simulink library "Renault_Library"  (Simulink 8.2)
settings: Solver=ode3  StartTime=0.0  StopTime=10.0  FixedStep=0.020  RelTol=1e-3
undefined symbols (not defined in the file or in its folder): ABC, AssystemProposal, b, chkval, CoeffA_C, CoeffB_C, CoeffMean_C, ConfTime_C, DiagDelay_C, DividePercent, FailureConfActive_C, FallingEdgeDuration_C, HighLevelThresholdVoltage_C, HighLevelVoltage_C, HighLevelVoltageTunable_C, InitValue_C, InputVoltRangeMax_C, InputVoltRangeMin_C, LowActiveContact_C, LowLevelThresholdVoltage_C, LowLevelVoltage_C, MaxOperatingVoltage_C, MinOperatingVoltage_C, mV2V_conversion, OUT1, OutOfRangeHigh_C, OutOfRangeLow_C, PinSupplySaturationMax, PinSupplySaturationMin, Res1Value_C, Res2Value_C, Res3Value_C, RisingEdgeDuration_C, RSA_CANSignalFailMonitoring, SampleTime_C, service14Table, service19Table, service22Table, service24Table, service28Table, service2ETable, service2FTable, service31Table, service87Table, SizeXStateDID2FTable, SizeXTable14, SizeXTable19, SizeXTable22, SizeXTable24, SizeXTable28, SizeXTable2E, SizeXTable2F, SizeXTable31, SizeXTable87, SizeYStateDID2FTable, SizeYTable14, SizeYTable19, SizeYTable22, SizeYTable24, SizeYTable28 ... (+22)
undefined symbols (not defined in the file): BatteryVoltage_ActivationThreshold_C, FrameStatus_AbsentValue_C, FrameStatus_ConfAbsentValue_C, MemThreshold_C, NAMEOFTHEMODEL_SAMPLE_TIME_CST, NbAbs1_C, NbConfAbs1_C, NbConfPres1_C, NbPres1_C, NOMDUSIGNAL_FaultConfTime_C, NOMDUSIGNAL_FaultRehabTime_C, NOMDUSIGNAL_switchvalue_C, NOMDUSIGNAL_unavailablevalue_C, PeriodFrame1_C, ResetTime_C, WakeUpTime_C
libraries: Renault_Library x112, simulink x75
(part 13 of 32)

== system Renault_Library/Services ECU/Diag/RSA_DiagnosticManagement_v02.00 ==
  DTOOL_TO_ECU_RECEIVED : Inport  OutDataTypeStr=uint8
  PART1_DTOOL_TO_ECU : Inport  Port=2  OutDataTypeStr=uint32
  PART2_DTOOL_TO_ECU : Inport  Port=3  OutDataTypeStr=uint32
  DIAG_INPUT : Inport  Port=4  OutDataTypeStr=Bus: busInput
  DIAGNOSTIC_ACTION_TOP : Subsystem
  DIAGNOSTIC_SANCTION_TOP : Subsystem
  DIAGNOSTIC_SEGMENTATION_TOP : Subsystem
  ECU_TO_DTOOL_RECEIVED : Outport  OutDataTypeStr=uint8
  PART1_ECU_TO_DTOOL : Outport  Port=2  OutDataTypeStr=uint32
  PART2_ECU_TO_DTOOL : Outport  Port=3  OutDataTypeStr=uint32
  DIAG_OUTPUT : Outport  Port=4  OutDataTypeStr=Bus: busOutput
  wires:
  DIAGNOSTIC_SEGMENTATION_TOP/2(PART1_ECU_TO_DTOOL) -> PART1_ECU_TO_DTOOL/1
  DIAGNOSTIC_SEGMENTATION_TOP/3(PART2_ECU_TO_DTOOL) -> PART2_ECU_TO_DTOOL/1
  DIAGNOSTIC_SEGMENTATION_TOP/1(ECU_TO_DTOOL_SEND) -> ECU_TO_DTOOL_RECEIVED/1
  [DIAG_BUFFER_INPUT] -> DIAGNOSTIC_SEGMENTATION_TOP/4(DIAG_BUFFER_RESPONSE)
  DIAGNOSTIC_ACTION_TOP/2(DIAG_BUFFER_RESPONSE) -> [DIAG_BUFFER_INPUT]
  DTOOL_TO_ECU_RECEIVED/1 -> DIAGNOSTIC_SEGMENTATION_TOP/1(DTOOL_TO_ECU_FRAME_RECEIVED)
  PART1_DTOOL_TO_ECU/1 -> DIAGNOSTIC_SEGMENTATION_TOP/2(PART1_DTOOL_TO_ECU)
  PART2_DTOOL_TO_ECU/1 -> DIAGNOSTIC_SEGMENTATION_TOP/3(PART2_DTOOL_TO_ECU)
  DIAG_INPUT/1 -> DIAGNOSTIC_ACTION_TOP/6(DIAG_INPUT)
  DIAGNOSTIC_ACTION_TOP/3(RES_DATA_NUMBER) -> [RES_DATA_NUMBER]
  [RES_DATA_NUMBER] -> DIAGNOSTIC_SEGMENTATION_TOP/5(RES_DATA_NUMBER)
  DIAGNOSTIC_SEGMENTATION_TOP/5(REQ_DATA_NUMBER) -> DIAGNOSTIC_SANCTION_TOP/2(REQ_DATA_NUMBER)
  DIAGNOSTIC_SEGMENTATION_TOP/6(SEG_STATE) -> DIAGNOSTIC_SANCTION_TOP/3(SEG_STATE)
  DIAGNOSTIC_SANCTION_TOP/1(NEGATIVE_RES_CODE) -> DIAGNOSTIC_ACTION_TOP/2(NEGATIVE_RES_CODE)
  DIAGNOSTIC_SANCTION_TOP/2(SUPPRESS_POS_RES) -> DIAGNOSTIC_ACTION_TOP/3(SUPPRESS_POS_RES)
  DIAGNOSTIC_SANCTION_TOP/3(SEND_RES_MESSAGE) -> DIAGNOSTIC_ACTION_TOP/4(SEND_RES_MESSAGE)
  DIAGNOSTIC_SANCTION_TOP/4(LIST_DID_SERVICE_22) -> DIAGNOSTIC_ACTION_TOP/5(LIST_DID_SERVICE_22)
  DIAGNOSTIC_SEGMENTATION_TOP/4(DIAG_BUFFER_REQUEST) -> DIAGNOSTIC_ACTION_TOP/1(DIAG_BUFFER_REQUEST), DIAGNOSTIC_SANCTION_TOP/1(DIAG_BUFFER_REQUEST)
  DIAGNOSTIC_ACTION_TOP/1(DIAG_OUTPUT) -> DIAG_OUTPUT/1

== system Renault_Library/Services ECU/Diag/RSA_FailureManagementSwitch ==
  FunctionalSignal : Inport
  Vbat : Inport  Port=2
  SignalFailure : Inport  Port=3
  SignalValue : Inport  Port=4
  SignalApplyValue : Inport  Port=5
  SignalFailureType : Inport  Port=6
  GroundValue : Constant  Value=GroundValue
  MultiportSwitch : MultiPortSwitch  Inputs=6  RndMeth=Zero  InputSameDT=on
  OpenCircuitValue : Constant  Value=OpenCircuitValue
  Switch : Switch  Criteria=u2 ~= 0  Threshold=0.5  RndMeth=Zero  InputSameDT=on
  Switch1 : Switch  Criteria=u2 ~= 0  Threshold=0.5  RndMeth=Zero  InputSameDT=on
  UnitDelay : UnitDelay  SampleTime=-1
  UnitDelay1 : UnitDelay  SampleTime=-1
  SignalOut : Outport
  wires:
  MultiportSwitch/1 -> Switch1/1
  SignalFailureType/1 -> MultiportSwitch/1
  Switch/1 -> UnitDelay/1, MultiportSwitch/4
  UnitDelay/1 -> Switch/3
  SignalFailure/1 -> Switch1/2
  FunctionalSignal/1 -> [FunctionalSignal]
  [FunctionalSignal] -> Switch1/3
  Switch1/1 -> SignalOut/1, [SignalOut]
  [FunctionalSignal] -> MultiportSwitch/2
  SignalValue/1 -> Switch/1
  [SignalOut] -> UnitDelay1/1
  UnitDelay1/1 -> MultiportSwitch/3
  GroundValue/1 -> MultiportSwitch/5
  OpenCircuitValue/1 -> MultiportSwitch/7
  Vbat/1 -> MultiportSwitch/6
  SignalApplyValue/1 -> Switch/2

== system Renault_Library/Services ECU/EEPROM/RSA_UpdateEEPROMData ==
  DATA_MODEL_EEPROM : Inport
      settings: AttributesFormatString=Type : %<OutDataTypeStr>
  DATA_STORE_READ : Inport  Port=2
      settings: AttributesFormatString=Type : %<OutDataTypeStr>
  RelationalOperator : RelationalOperator  Operator=~=  OutDataTypeStr=boolean  InputSameDT=on
  Switch : Switch  Criteria=u2 ~= 0  RndMeth=Zero  InputSameDT=on
      settings: AttributesFormatString=Threshold : %<Threshold>\nSaturation:%<SaturateOnIntegerOverflow>\nCriteria:%<Criteria>
  UnitDelay : UnitDelay  SampleTime=-1
      settings: AttributesFormatString=Initial value : %<X0>
  DATA_STORE_WRITE : Outport  OutputWhenDisabled=reset  InitialOutput=0
      settings: AttributesFormatString=Type : %<OutDataTypeStr>\nInit :%<InitialOutput>
  wires:
  DATA_MODEL_EEPROM/1 -> RelationalOperator/2, Switch/1, UnitDelay/1
  UnitDelay/1 -> RelationalOperator/1
  RelationalOperator/1 -> Switch/2
  DATA_STORE_READ/1 -> Switch/3
  Switch/1 -> DATA_STORE_WRITE/1

== system Renault_Library/Services ECU/HW Inputs/RSA_CHA8PRO ==
  note: cf. Modeling_Requirements_36-00-002_v1.2.doc
      | Requirements linked to this component:
      | RQT_LOGIN_GEN001_CHA8PRO
      | RQT_LOGIN_GEN002_CHA8PRO
      | RQT_LOGIN_GEN003_CHA8PRO
      | RQT_LOGIN_GEN004_CHA8PRO
      | RQT_LOGIN_GEN005_CHA8PRO
      | RQT_LOGIN_GEN006_CHA8PRO
      | RQT_LOGIN_GEN007_CHA8PRO
      | RQT_LOGIN_GEN008_CHA8PRO
  SwitchVoltage : Inport
  SupplyVoltage : Inport  Port=2
  RSA_ServECU_Activation : Renault_Library/Services ECU/Commonly_Used_Blocks/RSA_ServECU_Activation
  RSA_ServECU_EnabledSwitch : Renault_Library/Services ECU/Commonly_Used_Blocks/RSA_ServECU_EnabledSwitch
  Terminator : Terminator
  LogicalState_F : Outport  OutputWhenDisabled=reset  InitialOutput=0
  wires:
  SwitchVoltage/1 -> RSA_ServECU_EnabledSwitch/1
  RSA_ServECU_EnabledSwitch/1 -> Terminator/1
  RSA_ServECU_EnabledSwitch/2 -> LogicalState_F/1
  SupplyVoltage/1 -> RSA_ServECU_Activation/1
  RSA_ServECU_Activation/1 -> RSA_ServECU_EnabledSwitch/Enable

== system Renault_Library/Services ECU/HW Inputs/RSA_CHB6 ==
  note: cf. Modeling_Requirements_36-00-002_v1.2.doc
      | Requirements linked to this component:
      | RQT_LOGIN_GEN001_CHB6
      | RQT_LOGIN_GEN002_CHB6
      | RQT_LOGIN_GEN003_CHB6
      | RQT_LOGIN_GEN004_CHB6
      | RQT_LOGIN_GEN005_CHB6
      | RQT_LOGIN_GEN006_CHB6
      | RQT_LOGIN_GEN007_CHB6
      | RQT_LOGIN_GEN008_CHB6
  SwitchVoltage : Inport
  SupplyVoltage : Inport  Port=2
  RSA_ServECU_Activation : Renault_Library/Services ECU/Commonly_Used_Blocks/RSA_ServECU_Activation
  RSA_ServECU_EnabledSwitch : Renault_Library/Services ECU/Commonly_Used_Blocks/RSA_ServECU_EnabledSwitch
  Terminator : Terminator
  LogicalState_F : Outport  OutputWhenDisabled=reset  InitialOutput=0
  wires:
  RSA_ServECU_Activation/1 -> RSA_ServECU_EnabledSwitch/Enable
  SupplyVoltage/1 -> RSA_ServECU_Activation/1
  SwitchVoltage/1 -> RSA_ServECU_EnabledSwitch/1
  RSA_ServECU_EnabledSwitch/1 -> Terminator/1
  RSA_ServECU_EnabledSwitch/2 -> LogicalState_F/1

== system Renault_Library/Services ECU/HW Inputs/RSA_CHB6DAS ==
  note: cf. Modeling_Requirements_36-00-002_v1.2.doc
      | Requirements linked to this component:
      | RQT_LOGIN_GEN001_CHB6DAS
      | RQT_LOGIN_GEN002_CHB6DAS
      | RQT_LOGIN_GEN003_CHB6DAS
      | RQT_LOGIN_GEN004_CHB6DAS
      | RQT_LOGIN_GEN005_CHB6DAS
      | RQT_LOGIN_GEN006_CHB6DAS
      | RQT_LOGIN_GEN007_CHB6DAS
      | RQT_LOGIN_GEN008_CHB6DAS
  SwitchVoltage : Inport
  SupplyVoltage : Inport  Port=2
  RSA_ServECU_Activation : Renault_Library/Services ECU/Commonly_Used_Blocks/RSA_ServECU_Activation
  RSA_ServECU_EnabledSwitch : Renault_Library/Services ECU/Commonly_Used_Blocks/RSA_ServECU_EnabledSwitch
  Terminator : Terminator
  LogicalState_F : Outport  OutputWhenDisabled=reset  InitialOutput=0
  wires:
  SwitchVoltage/1 -> RSA_ServECU_EnabledSwitch/1
  RSA_ServECU_EnabledSwitch/1 -> Terminator/1
  RSA_ServECU_EnabledSwitch/2 -> LogicalState_F/1
  RSA_ServECU_Activation/1 -> RSA_ServECU_EnabledSwitch/Enable
  SupplyVoltage/1 -> RSA_ServECU_Activation/1

== system Renault_Library/Services ECU/HW Inputs/RSA_CHXX ==
  note: cf. Modeling_Requirements_36-00-002_v1.2.doc
      | Requirements linked to this component:
      | RQT_LOGIN_GEN001_CHXX
      | RQT_LOGIN_GEN002_CHXX
      | RQT_LOGIN_GEN003_CHXX
      | RQT_LOGIN_GEN004_CHXX
      | RQT_LOGIN_GEN005_CHXX
      | RQT_LOGIN_GEN006_CHXX
      | RQT_LOGIN_GEN007_CHXX
      | RQT_LOGIN_GEN008_CHXX
  SwitchVoltage : Inport
  SupplyVoltage : Inport  Port=2
  RSA_ServECU_Activation : Renault_Library/Services ECU/Commonly_Used_Blocks/RSA_ServECU_Activation
  RSA_ServECU_EnabledSwitch : Renault_Library/Services ECU/Commonly_Used_Blocks/RSA_ServECU_EnabledSwitch
  Terminator : Terminator
  LogicalState_F : Outport  OutputWhenDisabled=reset  InitialOutput=0
  wires:
  RSA_ServECU_EnabledSwitch/2 -> LogicalState_F/1
  RSA_ServECU_EnabledSwitch/1 -> Terminator/1
  SwitchVoltage/1 -> RSA_ServECU_EnabledSwitch/1
  SupplyVoltage/1 -> RSA_ServECU_Activation/1
  RSA_ServECU_Activation/1 -> RSA_ServECU_EnabledSwitch/Enable

== system Renault_Library/Services ECU/HW Inputs/RSA_CLA8LOCK ==
  note: cf. Modeling_Requirements_36-00-002_v1.2.doc
      | Requirements linked to this component:
      | RQT_ALOGIN_GEN001_CLA8LOCK
      | RQT_ALOGIN_GEN002_CLA8LOCK
      | RQT_ALOGIN_GEN003_CLA8LOCK
      | RQT_ALOGIN_GEN004_CLA8LOCK
      | RQT_ALOGIN_GEN005_CLA8LOCK
      | RQT_ALOGIN_GEN006_CLA8LOCK
      | RQT_ALOGIN_GEN007_CLA8LOCK
      | RQT_ALOGIN_GEN008_CLA8LOCK
      | RQT_ALOGIN_GEN009_CLA8LOCK
  SwitchVoltage : Inport
  SupplyVoltage : Inport  Port=2
  RSA_ServECU_Activation : Renault_Library/Services ECU/Commonly_Used_Blocks/RSA_ServECU_Activation
  RSA_ServECU_EnabledInputContact : Renault_Library/Services ECU/Commonly_Used_Blocks/RSA_ServECU_EnabledInputContact
  Terminator : Terminator
  SwitchState_F : Outport
  wires:
  SwitchVoltage/1 -> RSA_ServECU_EnabledInputContact/1
  SupplyVoltage/1 -> RSA_ServECU_Activation/1
  RSA_ServECU_EnabledInputContact/1 -> Terminator/1
  RSA_ServECU_EnabledInputContact/2 -> SwitchState_F/1
  RSA_ServECU_Activation/1 -> RSA_ServECU_EnabledInputContact/Enable

== system Renault_Library/Services ECU/HW Inputs/RSA_CLA8MVAC ==
  note: cf. Modeling_Requirements_36-00-002_v1.2.doc
      | Requirements linked to this component:
      | RQT_ALOGIN_GEN001_CLA8MVAC
      | RQT_ALOGIN_GEN002_CLA8MVAC
      | RQT_ALOGIN_GEN003_CLA8MVAC
      | RQT_ALOGIN_GEN004_CLA8MVAC
      | RQT_ALOGIN_GEN005_CLA8MVAC
      | RQT_ALOGIN_GEN006_CLA8MVAC
      | RQT_ALOGIN_GEN007_CLA8MVAC
      | RQT_ALOGIN_GEN008_CLA8MVAC
      | RQT_ALOGIN_GEN009_CLA8MVAC
  SwitchVoltage : Inport
  SupplyVoltage : Inport  Port=2
  RSA_ServECU_Activation : Renault_Library/Services ECU/Commonly_Used_Blocks/RSA_ServECU_Activation
  RSA_ServECU_EnabledInputContact : Renault_Library/Services ECU/Commonly_Used_Blocks/RSA_ServECU_EnabledInputContact
  Terminator : Terminator
  SwitchState_F : Outport
  wires:
  RSA_ServECU_Activation/1 -> RSA_ServECU_EnabledInputContact/Enable
  RSA_ServECU_EnabledInputContact/2 -> SwitchState_F/1
  RSA_ServECU_EnabledInputContact/1 -> Terminator/1
  SupplyVoltage/1 -> RSA_ServECU_Activation/1
  SwitchVoltage/1 -> RSA_ServECU_EnabledInputContact/1

== system Renault_Library/Services ECU/HW Inputs/RSA_CLA8PRO ==
  note: cf. Modeling_Requirements_36-00-002_v1.2.doc
      | Requirements linked to this component:
      | RQT_LOGIN_GEN001_CLA8PRO
      | RQT_LOGIN_GEN002_CLA8PRO
      | RQT_LOGIN_GEN003_CLA8PRO
      | RQT_LOGIN_GEN004_CLA8PRO
      | RQT_LOGIN_GEN005_CLA8PRO
      | RQT_LOGIN_GEN006_CLA8PRO
      | RQT_LOGIN_GEN007_CLA8PRO
      | RQT_LOGIN_GEN008_CLA8PRO
  SwitchVoltage : Inport
  SupplyVoltage : Inport  Port=2
  RSA_ServECU_Activation : Renault_Library/Services ECU/Commonly_Used_Blocks/RSA_ServECU_Activation
  RSA_ServECU_EnabledSwitch : Renault_Library/Services ECU/Commonly_Used_Blocks/RSA_ServECU_EnabledSwitch
  Terminator : Terminator
  LogicalState_F : Outport  OutputWhenDisabled=reset  InitialOutput=0
  wires:
  SupplyVoltage/1 -> RSA_ServECU_Activation/1
  SwitchVoltage/1 -> RSA_ServECU_EnabledSwitch/1
  RSA_ServECU_Activation/1 -> RSA_ServECU_EnabledSwitch/Enable
  RSA_ServECU_EnabledSwitch/1 -> Terminator/1
  RSA_ServECU_EnabledSwitch/2 -> LogicalState_F/1

== system Renault_Library/Services ECU/HW Inputs/RSA_CLA8SEAT ==
  note: cf. Modeling_Requirements_36-00-002_v1.2.doc
      | Requirements linked to this component:
      | RQT_ALOGIN_GEN001_CLA8SEAT
      | RQT_ALOGIN_GEN002_CLA8SEAT
      | RQT_ALOGIN_GEN003_CLA8SEAT
      | RQT_ALOGIN_GEN004_CLA8SEAT
      | RQT_ALOGIN_GEN005_CLA8SEAT
      | RQT_ALOGIN_GEN006_CLA8SEAT
      | RQT_ALOGIN_GEN007_CLA8SEAT
      | RQT_ALOGIN_GEN008_CLA8SEAT
      | RQT_ALOGIN_GEN009_CLA8SEAT
  SwitchVoltage : Inport
  SupplyVoltage : Inport  Port=2
  RSA_ServECU_Activation : Renault_Library/Services ECU/Commonly_Used_Blocks/RSA_ServECU_Activation
  RSA_ServECU_EnabledInputContact : Renault_Library/Services ECU/Commonly_Used_Blocks/RSA_ServECU_EnabledInputContact
  Terminator : Terminator
  SwitchState_F : Outport
  wires:
  RSA_ServECU_Activation/1 -> RSA_ServECU_EnabledInputContact/Enable
  RSA_ServECU_EnabledInputContact/2 -> SwitchState_F/1
  RSA_ServECU_EnabledInputContact/1 -> Terminator/1
  SupplyVoltage/1 -> RSA_ServECU_Activation/1
  SwitchVoltage/1 -> RSA_ServECU_EnabledInputContact/1

== system Renault_Library/Services ECU/HW Inputs/RSA_CLA8WAS ==
  note: cf. Modeling_Requirements_36-00-002_v1.2.doc
      | Requirements linked to this component:
      | RQT_LOGIN_GEN001_CLA8WAS
      | RQT_LOGIN_GEN002_CLA8WAS
      | RQT_LOGIN_GEN003_CLA8WAS
      | RQT_LOGIN_GEN004_CLA8WAS
      | RQT_LOGIN_GEN005_CLA8WAS
      | RQT_LOGIN_GEN006_CLA8WAS
      | RQT_LOGIN_GEN007_CLA8WAS
      | RQT_LOGIN_GEN008_CLA8WAS
  SwitchVoltage : Inport
  SupplyVoltage : Inport  Port=2
  RSA_ServECU_Activation : Renault_Library/Services ECU/Commonly_Used_Blocks/RSA_ServECU_Activation
  RSA_ServECU_EnabledSwitch : Renault_Library/Services ECU/Commonly_Used_Blocks/RSA_ServECU_EnabledSwitch
  Terminator : Terminator
  LogicalState_F : Outport  OutputWhenDisabled=reset  InitialOutput=0
  wires:
  RSA_ServECU_EnabledSwitch/2 -> LogicalState_F/1
  RSA_ServECU_EnabledSwitch/1 -> Terminator/1
  RSA_ServECU_Activation/1 -> RSA_ServECU_EnabledSwitch/Enable
  SupplyVoltage/1 -> RSA_ServECU_Activation/1
  SwitchVoltage/1 -> RSA_ServECU_EnabledSwitch/1

== system Renault_Library/Services ECU/HW Inputs/RSA_CLB6 ==
  note: cf. Modeling_Requirements_36-00-002_v1.2.doc
      | Requirements linked to this component:
      | RQT_LOGIN_GEN001_CLB6
      | RQT_LOGIN_GEN002_CLB6
      | RQT_LOGIN_GEN003_CLB6
      | RQT_LOGIN_GEN004_CLB6
      | RQT_LOGIN_GEN005_CLB6
      | RQT_LOGIN_GEN006_CLB6
      | RQT_LOGIN_GEN007_CLB6
      | RQT_LOGIN_GEN008_CLB6
  SwitchVoltage : Inport
  SupplyVoltage : Inport  Port=2
  RSA_ServECU_Activation : Renault_Library/Services ECU/Commonly_Used_Blocks/RSA_ServECU_Activation
  RSA_ServECU_EnabledSwitch : Renault_Library/Services ECU/Commonly_Used_Blocks/RSA_ServECU_EnabledSwitch
  Terminator : Terminator
  LogicalState_F : Outport  OutputWhenDisabled=reset  InitialOutput=0
  wires:
  RSA_ServECU_EnabledSwitch/2 -> LogicalState_F/1
  RSA_ServECU_EnabledSwitch/1 -> Terminator/1
  RSA_ServECU_Activation/1 -> RSA_ServECU_EnabledSwitch/Enable
  SwitchVoltage/1 -> RSA_ServECU_EnabledSwitch/1
  SupplyVoltage/1 -> RSA_ServECU_Activation/1

== system Renault_Library/Services ECU/HW Inputs/RSA_CLB6DAS ==
  note: cf. Modeling_Requirements_36-00-002_v1.2.doc
      | Requirements linked to this component:
      | RQT_LOGIN_GEN001_CLB6DAS
      | RQT_LOGIN_GEN002_CLB6DAS
      | RQT_LOGIN_GEN003_CLB6DAS
      | RQT_LOGIN_GEN004_CLB6DAS
      | RQT_LOGIN_GEN005_CLB6DAS
      | RQT_LOGIN_GEN006_CLB6DAS
      | RQT_LOGIN_GEN007_CLB6DAS
      | RQT_LOGIN_GEN008_CLB6DAS
  SwitchVoltage : Inport
  SupplyVoltage : Inport  Port=2
  RSA_ServECU_Activation : Renault_Library/Services ECU/Commonly_Used_Blocks/RSA_ServECU_Activation
  RSA_ServECU_EnabledSwitch : Renault_Library/Services ECU/Commonly_Used_Blocks/RSA_ServECU_EnabledSwitch
  Terminator : Terminator
  LogicalState_F : Outport  OutputWhenDisabled=reset  InitialOutput=0
  wires:
  SwitchVoltage/1 -> RSA_ServECU_EnabledSwitch/1
  RSA_ServECU_EnabledSwitch/1 -> Terminator/1
  RSA_ServECU_Activation/1 -> RSA_ServECU_EnabledSwitch/Enable
  SupplyVoltage/1 -> RSA_ServECU_Activation/1
  RSA_ServECU_EnabledSwitch/2 -> LogicalState_F/1

== system Renault_Library/Services ECU/HW Inputs/RSA_CLXX ==
  note: cf. Modeling_Requirements_36-00-002_v1.2.doc
      | Requirements linked to this component:
      | RQT_LOGIN_GEN001_CLXX
      | RQT_LOGIN_GEN002_CLXX
      | RQT_LOGIN_GEN003_CLXX
      | RQT_LOGIN_GEN004_CLXX
      | RQT_LOGIN_GEN005_CLXX
      | RQT_LOGIN_GEN006_CLXX
      | RQT_LOGIN_GEN007_CLXX
      | RQT_LOGIN_GEN008_CLXX
  SwitchVoltage : Inport
  SupplyVoltage : Inport  Port=2
  RSA_ServECU_Activation : Renault_Library/Services ECU/Commonly_Used_Blocks/RSA_ServECU_Activation
  RSA_ServECU_EnabledSwitch : Renault_Library/Services ECU/Commonly_Used_Blocks/RSA_ServECU_EnabledSwitch
  Terminator : Terminator
  LogicalState_F : Outport  OutputWhenDisabled=reset  InitialOutput=0
  wires:
  SupplyVoltage/1 -> RSA_ServECU_Activation/1
  SwitchVoltage/1 -> RSA_ServECU_EnabledSwitch/1
  RSA_ServECU_Activation/1 -> RSA_ServECU_EnabledSwitch/Enable
  RSA_ServECU_EnabledSwitch/1 -> Terminator/1
  RSA_ServECU_EnabledSwitch/2 -> LogicalState_F/1

== system Renault_Library/Services ECU/HW Inputs/RSA_IHB6 ==
  note: cf. Modeling_Requirements_36-00-002_v1.2.doc
      | Requirements linked to this component:
      | RQT_LOGIN_GEN001_IHB6
      | RQT_LOGIN_GEN002_IHB6
      | RQT_LOGIN_GEN003_IHB6
      | RQT_LOGIN_GEN004_IHB6
      | RQT_LOGIN_GEN005_IHB6
      | RQT_LOGIN_GEN006_IHB6
      | RQT_LOGIN_GEN007_IHB6
      | RQT_LOGIN_GEN008_IHB6
  SwitchVoltage : Inport
  SupplyVoltage : Inport  Port=2
  RSA_ServECU_Activation : Renault_Library/Services ECU/Commonly_Used_Blocks/RSA_ServECU_Activation
  RSA_ServECU_EnabledSwitch : Renault_Library/Services ECU/Commonly_Used_Blocks/RSA_ServECU_EnabledSwitch
  Terminator : Terminator
  LogicalState_F : Outport  OutputWhenDisabled=reset  InitialOutput=0
  wires:
  SupplyVoltage/1 -> RSA_ServECU_Activation/1
  SwitchVoltage/1 -> RSA_ServECU_EnabledSwitch/1
  RSA_ServECU_Activation/1 -> RSA_ServECU_EnabledSwitch/Enable
  RSA_ServECU_EnabledSwitch/1 -> Terminator/1
  RSA_ServECU_EnabledSwitch/2 -> LogicalState_F/1

== system Renault_Library/Services ECU/HW Inputs/RSA_IHB6LED ==
  note: cf. Modeling_Requirements_36-00-002_v1.2.doc
      | Requirements linked to this component:
      | RQT_LOGIN_GEN001_IHB6LED
      | RQT_LOGIN_GEN002_IHB6LED
      | RQT_LOGIN_GEN003_IHB6LED
      | RQT_LOGIN_GEN004_IHB6LED
      | RQT_LOGIN_GEN005_IHB6LED
      | RQT_LOGIN_GEN006_IHB6LED
      | RQT_LOGIN_GEN007_IHB6LED
      | RQT_LOGIN_GEN008_IHB6LED
      | RQT_LOGIN_ADDGEN001_IHB6LED
  SwitchVoltage : Inport
  SupplyVoltage : Inport  Port=2
  RSA_ServECU_Activation : Renault_Library/Services ECU/Commonly_Used_Blocks/RSA_ServECU_Activation
  RSA_ServECU_EnabledSwitch : Renault_Library/Services ECU/Commonly_Used_Blocks/RSA_ServECU_EnabledSwitch
  LogicalState_LED : Outport  OutputWhenDisabled=reset  InitialOutput=0
  LogicalState_F : Outport  Port=2  OutputWhenDisabled=reset  InitialOutput=0
  wires:
  RSA_ServECU_EnabledSwitch/2 -> LogicalState_F/1
  RSA_ServECU_EnabledSwitch/1 -> LogicalState_LED/1
  RSA_ServECU_Activation/1 -> RSA_ServECU_EnabledSwitch/Enable
  SwitchVoltage/1 -> RSA_ServECU_EnabledSwitch/1
  SupplyVoltage/1 -> RSA_ServECU_Activation/1

== system Renault_Library/Services ECU/HW Inputs/RSA_IHXX ==
  note: cf. Modeling_Requirements_36-00-002_v1.2.doc
      | Requirements linked to this component:
      | RQT_LOGIN_GEN001_IHXX
      | RQT_LOGIN_GEN002_IHXX
      | RQT_LOGIN_GEN003_IHXX
      | RQT_LOGIN_GEN004_IHXX
      | RQT_LOGIN_GEN005_IHXX
      | RQT_LOGIN_GEN006_IHXX
      | RQT_LOGIN_GEN007_IHXX
      | RQT_LOGIN_GEN008_IHXX
  SwitchVoltage : Inport
  SupplyVoltage : Inport  Port=2
  RSA_ServECU_Activation : Renault_Library/Services ECU/Commonly_Used_Blocks/RSA_ServECU_Activation
  RSA_ServECU_EnabledSwitch : Renault_Library/Services ECU/Commonly_Used_Blocks/RSA_ServECU_EnabledSwitch
  Terminator : Terminator
  LogicalState_F : Outport  OutputWhenDisabled=reset  InitialOutput=0
  wires:
  SupplyVoltage/1 -> RSA_ServECU_Activation/1
  SwitchVoltage/1 -> RSA_ServECU_EnabledSwitch/1
  RSA_ServECU_Activation/1 -> RSA_ServECU_EnabledSwitch/Enable
  RSA_ServECU_EnabledSwitch/1 -> Terminator/1
  RSA_ServECU_EnabledSwitch/2 -> LogicalState_F/1

== system Renault_Library/Services ECU/HW Inputs/RSA_ILB6 ==
  note: cf. Modeling_Requirements_36-00-002_v1.2.doc
      | Requirements linked to this component:
      | RQT_LOGIN_GEN001_IHB6
      | RQT_LOGIN_GEN002_IHB6
      | RQT_LOGIN_GEN003_IHB6
      | RQT_LOGIN_GEN004_IHB6
      | RQT_LOGIN_GEN005_IHB6
      | RQT_LOGIN_GEN006_IHB6
      | RQT_LOGIN_GEN007_IHB6
      | RQT_LOGIN_GEN008_IHB6
  SwitchVoltage : Inport
  SupplyVoltage : Inport  Port=2
  RSA_ServECU_Activation : Renault_Library/Services ECU/Commonly_Used_Blocks/RSA_ServECU_Activation
  RSA_ServECU_EnabledSwitch : Renault_Library/Services ECU/Commonly_Used_Blocks/RSA_ServECU_EnabledSwitch
  Terminator : Terminator
  LogicalState_F : Outport  OutputWhenDisabled=reset  InitialOutput=0
  wires:
  SupplyVoltage/1 -> RSA_ServECU_Activation/1
  SwitchVoltage/1 -> RSA_ServECU_EnabledSwitch/1
  RSA_ServECU_Activation/1 -> RSA_ServECU_EnabledSwitch/Enable
  RSA_ServECU_EnabledSwitch/1 -> Terminator/1
  RSA_ServECU_EnabledSwitch/2 -> LogicalState_F/1

== system Renault_Library/Services ECU/HW Inputs/RSA_ILB6LED ==
  note: cf. Modeling_Requirements_36-00-002_v1.2.doc
      | Requirements linked to this component:
      | RQT_LOGIN_GEN001_ILB6LED
      | RQT_LOGIN_GEN002_ILB6LED
      | RQT_LOGIN_GEN003_ILB6LED
      | RQT_LOGIN_GEN004_ILB6LED
      | RQT_LOGIN_GEN005_ILB6LED
      | RQT_LOGIN_GEN006_ILB6LED
      | RQT_LOGIN_GEN007_ILB6LED
      | RQT_LOGIN_GEN008_ILB6LED
      | RQT_LOGIN_ADDGEN001_ILB6LED
  SwitchVoltage : Inport
  SupplyVoltage : Inport  Port=2
  RSA_ServECU_Activation : Renault_Library/Services ECU/Commonly_Used_Blocks/RSA_ServECU_Activation
  RSA_ServECU_EnabledSwitch : Renault_Library/Services ECU/Commonly_Used_Blocks/RSA_ServECU_EnabledSwitch
  LogicalState_LED : Outport  OutputWhenDisabled=reset  InitialOutput=0
  LogicalState_F : Outport  Port=2  OutputWhenDisabled=reset  InitialOutput=0
  wires:
  SupplyVoltage/1 -> RSA_ServECU_Activation/1
  SwitchVoltage/1 -> RSA_ServECU_EnabledSwitch/1
  RSA_ServECU_Activation/1 -> RSA_ServECU_EnabledSwitch/Enable
  RSA_ServECU_EnabledSwitch/1 -> LogicalState_LED/1
  RSA_ServECU_EnabledSwitch/2 -> LogicalState_F/1

== system Renault_Library/Services ECU/HW Inputs/RSA_ILXX ==
  note: cf. Modeling_Requirements_36-00-002_v1.2.doc
      | Requirements linked to this component:
      | RQT_LOGIN_GEN001_ILXX
      | RQT_LOGIN_GEN002_ILXX
      | RQT_LOGIN_GEN003_ILXX
      | RQT_LOGIN_GEN004_ILXX
      | RQT_LOGIN_GEN005_ILXX
      | RQT_LOGIN_GEN006_ILXX
      | RQT_LOGIN_GEN007_ILXX
      | RQT_LOGIN_GEN008_ILXX
  SwitchVoltage : Inport
  SupplyVoltage : Inport  Port=2
  RSA_ServECU_Activation : Renault_Library/Services ECU/Commonly_Used_Blocks/RSA_ServECU_Activation
  RSA_ServECU_EnabledSwitch : Renault_Library/Services ECU/Commonly_Used_Blocks/RSA_ServECU_EnabledSwitch
  Terminator : Terminator
  LogicalState_F : Outport  OutputWhenDisabled=reset  InitialOutput=0
  wires:
  RSA_ServECU_EnabledSwitch/2 -> LogicalState_F/1
  RSA_ServECU_EnabledSwitch/1 -> Terminator/1
  RSA_ServECU_Activation/1 -> RSA_ServECU_EnabledSwitch/Enable
  SwitchVoltage/1 -> RSA_ServECU_EnabledSwitch/1
  SupplyVoltage/1 -> RSA_ServECU_Activation/1

== system Renault_Library/Services ECU/HW Inputs/RSA_IZB5TTL ==
  note: cf. Modeling_Requirements_36-00-002_v1.2.doc
      | Requirements linked to this component:
      | RQT_LOGIN_GEN001_IZB5TTL
      | RQT_LOGIN_GEN002_IZB5TTL
      | RQT_LOGIN_GEN003_IZB5TTL
      | RQT_LOGIN_GEN004_IZB5TTL
      | RQT_LOGIN_GEN005_IZB5TTL
      | RQT_LOGIN_GEN006_IZB5TTL
      | RQT_LOGIN_GEN007_IZB5TTL
      | RQT_LOGIN_GEN008_IZB5TTL
  SwitchVoltage : Inport
  SupplyVoltage : Inport  Port=2
  RSA_ServECU_Activation : Renault_Library/Services ECU/Commonly_Used_Blocks/RSA_ServECU_Activation
  RSA_ServECU_EnabledSwitch : Renault_Library/Services ECU/Commonly_Used_Blocks/RSA_ServECU_EnabledSwitch
  Terminator : Terminator
  LogicalState_F : Outport  OutputWhenDisabled=reset  InitialOutput=0
  wires:
  SupplyVoltage/1 -> RSA_ServECU_Activation/1
  SwitchVoltage/1 -> RSA_ServECU_EnabledSwitch/1
  RSA_ServECU_Activation/1 -> RSA_ServECU_EnabledSwitch/Enable
  RSA_ServECU_EnabledSwitch/1 -> Terminator/1
  RSA_ServECU_EnabledSwitch/2 -> LogicalState_F/1

== system Renault_Library/Services ECU/HW Inputs/RSA_RHEO9 ==
  note: cf. Modeling_Requirements_36-00-002_v1.2.doc
      | Requirements linked to this component:
      | RQT_ALOGIN_VOL_GEN001_RHEO9
      | RQT_ALOGIN_VOL_GEN002_RHEO9
      | RQT_ALOGIN_VOL_GEN003_RHEO9
      | RQT_ALOGIN_VOL_GEN004_RHEO9
      | RQT_ALOGIN_VOL_GEN005_RHEO9
      | RQT_ALOGIN_VOL_GEN006_RHEO9
      | RQT_ALOGIN_VOL_GEN007_RHEO9
  InputVoltage : Inport
  SupplyVoltage : Inport  Port=2
  RSA_ServECU_Activation : Renault_Library/Services ECU/Commonly_Used_Blocks/RSA_ServECU_Activation
  RSA_ServECU_EnabledSupply : Renault_Library/Services ECU/Commonly_Used_Blocks/RSA_ServECU_EnabledSupply
  Terminator : Terminator
  OutputVoltage_F : Outport  OutputWhenDisabled=reset  InitialOutput=0
  MeanOutputVoltage : Outport  Port=2  OutputWhenDisabled=reset  InitialOutput=0
  wires:
  SupplyVoltage/1 -> RSA_ServECU_Activation/1
  RSA_ServECU_Activation/1 -> RSA_ServECU_EnabledSupply/Enable
  RSA_ServECU_EnabledSupply/2 -> OutputVoltage_F/1
  RSA_ServECU_EnabledSupply/1 -> Terminator/1
  InputVoltage/1 -> RSA_ServECU_EnabledSupply/1
  RSA_ServECU_EnabledSupply/3 -> MeanOutputVoltage/1

== system Renault_Library/Services ECU/HW Inputs/RSA_VMEAS6 ==
  note: cf. Modeling_Requirements_36-00-002_v1.2.doc
      | Requirements linked to this component:
      | RQT_ALOGIN_VOL_GEN001_VMEAS6
      | RQT_ALOGIN_VOL_GEN002_VMEAS6
      | RQT_ALOGIN_VOL_GEN003_VMEAS6
      | RQT_ALOGIN_VOL_GEN004_VMEAS6
      | RQT_ALOGIN_VOL_GEN005_VMEAS6
      | RQT_ALOGIN_VOL_GEN006_VMEAS6
      | RQT_ALOGIN_VOL_GEN007_VMEAS6
  InputVoltage : Inport
  SupplyVoltage : Inport  Port=2
  RSA_ServECU_Activation : Renault_Library/Services ECU/Commonly_Used_Blocks/RSA_ServECU_Activation
  RSA_ServECU_EnabledSupply : Renault_Library/Services ECU/Commonly_Used_Blocks/RSA_ServECU_EnabledSupply
  Terminator : Terminator
  OutputVoltage_F : Outport  OutputWhenDisabled=reset  InitialOutput=0
  MeanOutputVoltage : Outport  Port=2  OutputWhenDisabled=reset  InitialOutput=0
  wires:
  RSA_ServECU_EnabledSupply/1 -> Terminator/1
  RSA_ServECU_EnabledSupply/2 -> OutputVoltage_F/1
  RSA_ServECU_Activation/1 -> RSA_ServECU_EnabledSupply/Enable
  SupplyVoltage/1 -> RSA_ServECU_Activation/1
  InputVoltage/1 -> RSA_ServECU_EnabledSupply/1
  RSA_ServECU_EnabledSupply/3 -> MeanOutputVoltage/1

== system Renault_Library/Services ECU/HW Outputs/RSA_IHAB6Y ==
  note: cf. Modeling_Requirements_36-00-002_v1.2.doc
      | Requirements linked to this component:
      | RQT_LOGOUT_GEN001_IHAB6Y
      | RQT_LOGOUT_GEN002_IHAB6Y
      | RQT_LOGOUT_GEN003_IHAB6Y
      | RQT_LOGOUT_GEN004_IHAB6Y
      | RQT_LOGOUT_GEN005_IHAB6Y
      | RQT_LOGOUT_GEN006_IHAB6Y
      | RQT_LOGOUT_GEN007_IHAB6Y
      | RQT_LOGOUT_ADDGEN001_IHAB6Y
  LogicalState : Inport
  SupplyVoltage : Inport  Port=2
  RSA_ServECU_Activation : Renault_Library/Services ECU/Commonly_Used_Blocks/RSA_ServECU_Activation
  RSA_ServECU_EnaOutContactSat : Renault_Library/Services ECU/Commonly_Used_Blocks/RSA_ServECU_EnaOutContactSat
  PhysicalOutput_F : Outport
  wires:
  SupplyVoltage/1 -> RSA_ServECU_EnaOutContactSat/1, RSA_ServECU_Activation/1
  RSA_ServECU_Activation/1 -> RSA_ServECU_EnaOutContactSat/Enable
  LogicalState/1 -> RSA_ServECU_EnaOutContactSat/2
  RSA_ServECU_EnaOutContactSat/1 -> PhysicalOutput_F/1

== system Renault_Library/Services ECU/HW Outputs/RSA_IHABD6Y ==
  note: cf. Modeling_Requirements_36-00-002_v1.2.doc
      | Requirements linked to this component:
      | RQT_LOGOUT_GEN001_IHABD6Y
      | RQT_LOGOUT_GEN002_IHABD6Y
      | RQT_LOGOUT_GEN003_IHABD6Y
      | RQT_LOGOUT_GEN004_IHABD6Y
      | RQT_LOGOUT_GEN005_IHABD6Y
      | RQT_LOGOUT_GEN006_IHABD6Y
      | RQT_LOGOUT_GEN007_IHABD6Y
      | RQT_LOGOUT_ADDGEN001_IHABD6Y
      | RQT_LOGOUT_ADDGEN003_IHABD6Y
      | RQT_LOGOUT_ADDGEN004_IHABD6Y
  LogicalState : Inport
  SupplyVoltage : Inport  Port=2
  OutputFeedback : Inport  Port=3
  RSA_ServECU_Activation : Renault_Library/Services ECU/Commonly_Used_Blocks/RSA_ServECU_Activation
  RSA_ServECU_EnaOutContactSat : Renault_Library/Services ECU/Commonly_Used_Blocks/RSA_ServECU_EnaOutContactSat
  RSA_ServECU_EnabledDiagnostic : Renault_Library/Services ECU/Commonly_Used_Blocks/RSA_ServECU_EnabledDiagnostic
  Terminator : Terminator
  PhysicalOutput_F : Outport
  Diagnostic : Outport  Port=2
  wires:
  SupplyVoltage/1 -> RSA_ServECU_Activation/1, RSA_ServECU_EnaOutContactSat/1
  RSA_ServECU_Activation/1 -> RSA_ServECU_EnaOutContactSat/Enable
  OutputFeedback/1 -> RSA_ServECU_EnabledDiagnostic/2
  RSA_ServECU_EnaOutContactSat/1 -> PhysicalOutput_F/1
  LogicalState/1 -> RSA_ServECU_EnaOutContactSat/2, RSA_ServECU_EnabledDiagnostic/1
  RSA_ServECU_EnabledDiagnostic/2 -> Diagnostic/1
  RSA_ServECU_EnabledDiagnostic/1 -> Terminator/1

== system Renault_Library/Services ECU/HW Outputs/RSA_IHABDXY ==
  note: cf. Modeling_Requirements_36-00-002_v1.2.doc
      | Requirements linked to this component:
      | RQT_LOGOUT_GEN001_IHABDXY
      | RQT_LOGOUT_GEN002_IHABDXY
      | RQT_LOGOUT_GEN003_IHABDXY
      | RQT_LOGOUT_GEN004_IHABDXY
      | RQT_LOGOUT_GEN005_IHABDXY
      | RQT_LOGOUT_GEN006_IHABDXY
      | RQT_LOGOUT_GEN007_IHABDXY
      | RQT_LOGOUT_ADDGEN001_IHABDXY
      | RQT_LOGOUT_ADDGEN003_IHABDXY
      | RQT_LOGOUT_ADDGEN004_IHABDXY
  LogicalState : Inport
  SupplyVoltage : Inport  Port=2
  OutputFeedback : Inport  Port=3
  RSA_ServECU_Activation : Renault_Library/Services ECU/Commonly_Used_Blocks/RSA_ServECU_Activation
  RSA_ServECU_EnaOutContactSat : Renault_Library/Services ECU/Commonly_Used_Blocks/RSA_ServECU_EnaOutContactSat
  RSA_ServECU_EnabledDiagnostic : Renault_Library/Services ECU/Commonly_Used_Blocks/RSA_ServECU_EnabledDiagnostic
  Terminator : Terminator
  PhysicalOutput_F : Outport
  Diagnostic : Outport  Port=2
  wires:
  RSA_ServECU_EnabledDiagnostic/1 -> Terminator/1
  RSA_ServECU_EnabledDiagnostic/2 -> Diagnostic/1
  LogicalState/1 -> RSA_ServECU_EnabledDiagnostic/1, RSA_ServECU_EnaOutContactSat/2
  RSA_ServECU_EnaOutContactSat/1 -> PhysicalOutput_F/1
  OutputFeedback/1 -> RSA_ServECU_EnabledDiagnostic/2
  RSA_ServECU_Activation/1 -> RSA_ServECU_EnaOutContactSat/Enable
  SupplyVoltage/1 -> RSA_ServECU_EnaOutContactSat/1, RSA_ServECU_Activation/1

== system Renault_Library/Services ECU/HW Outputs/RSA_ILAB6Y ==
  note: cf. Modeling_Requirements_36-00-002_v1.2.doc
      | Requirements linked to this component:
      | RQT_LOGOUT_GEN001_ILAB6Y
      | RQT_LOGOUT_GEN002_ILAB6Y
      | RQT_LOGOUT_GEN003_ILAB6Y
      | RQT_LOGOUT_GEN004_ILAB6Y
      | RQT_LOGOUT_GEN005_ILAB6Y
      | RQT_LOGOUT_GEN006_ILAB6Y
      | RQT_LOGOUT_GEN007_ILAB6Y
      | RQT_LOGOUT_ADDGEN002_ILAB6Y
  LogicalState : Inport
  SupplyVoltage : Inport  Port=2
  RSA_ServECU_Activation : Renault_Library/Services ECU/Commonly_Used_Blocks/RSA_ServECU_Activation
  RSA_ServECU_EnaOutContact : Renault_Library/Services ECU/Commonly_Used_Blocks/RSA_ServECU_EnaOutContact
  PhysicalOutput_F : Outport
  wires:
  RSA_ServECU_EnaOutContact/1 -> PhysicalOutput_F/1
  LogicalState/1 -> RSA_ServECU_EnaOutContact/2
  RSA_ServECU_Activation/1 -> RSA_ServECU_EnaOutContact/Enable
  SupplyVoltage/1 -> RSA_ServECU_EnaOutContact/1, RSA_ServECU_Activation/1

== system Renault_Library/Services ECU/HW Outputs/RSA_ILAB8250REL ==
  note: cf. Modeling_Requirements_36-00-002_v1.2.doc
      | Requirements linked to this component:
      | RQT_LOGOUT_GEN001_ILAB8250REL
      | RQT_LOGOUT_GEN002_ILAB8250REL
      | RQT_LOGOUT_GEN003_ILAB8250REL
      | RQT_LOGOUT_GEN004_ILAB8250REL
      | RQT_LOGOUT_GEN005_ILAB8250REL
      | RQT_LOGOUT_GEN006_ILAB8250REL
      | RQT_LOGOUT_GEN007_ILAB8250REL
      | RQT_LOGOUT_ADDGEN002_ILAB8250REL
  LogicalState : Inport
  SupplyVoltage : Inport  Port=2
  RSA_ServECU_Activation : Renault_Library/Services ECU/Commonly_Used_Blocks/RSA_ServECU_Activation
  RSA_ServECU_EnaOutContact : Renault_Library/Services ECU/Commonly_Used_Blocks/RSA_ServECU_EnaOutContact
  PhysicalOutput_F : Outport
  wires:
  LogicalState/1 -> RSA_ServECU_EnaOutContact/2
  SupplyVoltage/1 -> RSA_ServECU_Activation/1, RSA_ServECU_EnaOutContact/1
  RSA_ServECU_EnaOutContact/1 -> PhysicalOutput_F/1
  RSA_ServECU_Activation/1 -> RSA_ServECU_EnaOutContact/Enable

== system Renault_Library/Services ECU/HW Outputs/RSA_ILABD6Y ==
  note: cf. Modeling_Requirements_36-00-002_v1.2.doc
      | Requirements linked to this component:
      | RQT_LOGOUT_GEN001_ILABD6Y
      | RQT_LOGOUT_GEN002_ILABD6Y
      | RQT_LOGOUT_GEN003_ILABD6Y
      | RQT_LOGOUT_GEN004_ILABD6Y
      | RQT_LOGOUT_GEN005_ILABD6Y
      | RQT_LOGOUT_GEN006_ILABD6Y
      | RQT_LOGOUT_GEN007_ILABD6Y
      | RQT_LOGOUT_ADDGEN002_ILABD6Y
      | RQT_LOGOUT_ADDGEN003_ILABD6Y
      | RQT_LOGOUT_ADDGEN004_ILABD6Y
  LogicalState : Inport
  SupplyVoltage : Inport  Port=2
  OutputFeedback : Inport  Port=3
  RSA_ServECU_Activation : Renault_Library/Services ECU/Commonly_Used_Blocks/RSA_ServECU_Activation
  RSA_ServECU_EnaOutContact : Renault_Library/Services ECU/Commonly_Used_Blocks/RSA_ServECU_EnaOutContact
  RSA_ServECU_EnabledDiagnostic : Renault_Library/Services ECU/Commonly_Used_Blocks/RSA_ServECU_EnabledDiagnostic
  Terminator : Terminator
  PhysicalOutput_F : Outport
  Diagnostic : Outport  Port=2
  wires:
  RSA_ServECU_EnaOutContact/1 -> PhysicalOutput_F/1
  LogicalState/1 -> RSA_ServECU_EnabledDiagnostic/1, RSA_ServECU_EnaOutContact/2
  OutputFeedback/1 -> RSA_ServECU_EnabledDiagnostic/2
  RSA_ServECU_Activation/1 -> RSA_ServECU_EnaOutContact/Enable
  SupplyVoltage/1 -> RSA_ServECU_Activation/1, RSA_ServECU_EnaOutContact/1
  RSA_ServECU_EnabledDiagnostic/2 -> Diagnostic/1
  RSA_ServECU_EnabledDiagnostic/1 -> Terminator/1

== system Renault_Library/Services ECU/HW Outputs/RSA_ILABD8250REL ==
  note: cf. Modeling_Requirements_36-00-002_v1.2.doc
      | Requirements linked to this component:
      | RQT_LOGOUT_GEN001_ILABD8250REL
      | RQT_LOGOUT_GEN002_ILABD8250REL
      | RQT_LOGOUT_GEN003_ILABD8250REL
      | RQT_LOGOUT_GEN004_ILABD8250REL
      | RQT_LOGOUT_GEN005_ILABD8250REL
      | RQT_LOGOUT_GEN006_ILABD8250REL
      | RQT_LOGOUT_GEN007_ILABD8250REL
      | RQT_LOGOUT_ADDGEN002_ILABD8250REL
      | RQT_LOGOUT_ADDGEN003_ILABD8250REL
  LogicalState : Inport
  SupplyVoltage : Inport  Port=2
  OutputFeedback : Inport  Port=3
  RSA_ServECU_Activation : Renault_Library/Services ECU/Commonly_Used_Blocks/RSA_ServECU_Activation
  RSA_ServECU_EnaOutContact : Renault_Library/Services ECU/Commonly_Used_Blocks/RSA_ServECU_EnaOutContact
  RSA_ServECU_EnabledDiagnostic : Renault_Library/Services ECU/Commonly_Used_Blocks/RSA_ServECU_EnabledDiagnostic
  Terminator : Terminator
  PhysicalOutput_F : Outport
  Diagnostic : Outport  Port=2
  wires:
  OutputFeedback/1 -> RSA_ServECU_EnabledDiagnostic/2
  LogicalState/1 -> RSA_ServECU_EnaOutContact/2, RSA_ServECU_EnabledDiagnostic/1
  SupplyVoltage/1 -> RSA_ServECU_Activation/1, RSA_ServECU_EnaOutContact/1
  RSA_ServECU_EnaOutContact/1 -> PhysicalOutput_F/1
  RSA_ServECU_Activation/1 -> RSA_ServECU_EnaOutContact/Enable
  RSA_ServECU_EnabledDiagnostic/2 -> Diagnostic/1
  RSA_ServECU_EnabledDiagnostic/1 -> Terminator/1

== system Renault_Library/Services ECU/HW Outputs/RSA_ILABDXY ==
  note: cf. Modeling_Requirements_36-00-002_v1.2.doc
      | Requirements linked to this component:
      | RQT_LOGOUT_GEN001_ILABDXY
      | RQT_LOGOUT_GEN002_ILABDXY
      | RQT_LOGOUT_GEN003_ILABDXY
      | RQT_LOGOUT_GEN004_ILABDXY
      | RQT_LOGOUT_GEN005_ILABDXY
      | RQT_LOGOUT_GEN006_ILABDXY
      | RQT_LOGOUT_GEN007_ILABDXY
      | RQT_LOGOUT_ADDGEN002_ILABDXY
      | RQT_LOGOUT_ADDGEN003_ILABDXY
      | RQT_LOGOUT_ADDGEN004_ILABDXY
  LogicalState : Inport
  SupplyVoltage : Inport  Port=2
  OutputFeedback : Inport  Port=3
  RSA_ServECU_Activation : Renault_Library/Services ECU/Commonly_Used_Blocks/RSA_ServECU_Activation
  RSA_ServECU_EnaOutContact : Renault_Library/Services ECU/Commonly_Used_Blocks/RSA_ServECU_EnaOutContact
  RSA_ServECU_EnabledDiagnostic : Renault_Library/Services ECU/Commonly_Used_Blocks/RSA_ServECU_EnabledDiagnostic
  Terminator : Terminator
  PhysicalOutput_F : Outport
  Diagnostic : Outport  Port=2
  wires:
  RSA_ServECU_EnabledDiagnostic/1 -> Terminator/1
  RSA_ServECU_EnabledDiagnostic/2 -> Diagnostic/1
  SupplyVoltage/1 -> RSA_ServECU_EnaOutContact/1, RSA_ServECU_Activation/1
  RSA_ServECU_Activation/1 -> RSA_ServECU_EnaOutContact/Enable
  OutputFeedback/1 -> RSA_ServECU_EnabledDiagnostic/2
  LogicalState/1 -> RSA_ServECU_EnaOutContact/2, RSA_ServECU_EnabledDiagnostic/1
  RSA_ServECU_EnaOutContact/1 -> PhysicalOutput_F/1

== system Renault_Library/Services ECU/HW Outputs/RSA_ILCD6Y ==
  note: cf. Modeling_Requirements_36-00-002_v1.2.doc
      | Requirements linked to this component:
      | RQT_LOGOUT_GEN001_ILCD6Y
      | RQT_LOGOUT_GEN002_ILCD6Y
      | RQT_LOGOUT_GEN003_ILCD6Y
      | RQT_LOGOUT_GEN004_ILCD6Y
      | RQT_LOGOUT_GEN005_ILCD6Y
      | RQT_LOGOUT_GEN006_ILCD6Y
      | RQT_LOGOUT_GEN007_ILCD6Y
      | RQT_LOGOUT_ADDGEN002_ILCD6Y
      | RQT_LOGOUT_ADDGEN003_ILCD6Y
      | RQT_LOGOUT_ADDGEN004_ILCD6Y
      | RQT_LOGOUT_ADDGEN005_ILCD6Y
  LogicalState : Inport
  SupplyVoltage : Inport  Port=2
  RSA_ServECU_Activation : Renault_Library/Services ECU/Commonly_Used_Blocks/RSA_ServECU_Activation
  RSA_ServECU_EnaOutContact : Renault_Library/Services ECU/Commonly_Used_Blocks/RSA_ServECU_EnaOutContact
  RSA_ServECU_EnabledDiagnostic : Renault_Library/Services ECU/Commonly_Used_Blocks/RSA_ServECU_EnabledDiagnostic
  Terminator : Terminator
  PhysicalOutput_F : Outport
  InternalFeedback : Outport  Port=2
  wires:
  SupplyVoltage/1 -> RSA_ServECU_Activation/1, RSA_ServECU_EnaOutContact/1
  RSA_ServECU_Activation/1 -> RSA_ServECU_EnaOutContact/Enable
  [OutputFeedback] -> RSA_ServECU_EnabledDiagnostic/2
  RSA_ServECU_EnabledDiagnostic/2 -> Terminator/1
  LogicalState/1 -> RSA_ServECU_EnabledDiagnostic/1, RSA_ServECU_EnaOutContact/2
  RSA_ServECU_EnaOutContact/1 -> PhysicalOutput_F/1, [OutputFeedback]
  RSA_ServECU_EnabledDiagnostic/1 -> InternalFeedback/1

== system Renault_Library/Services ECU/HW Outputs/RSA_IZCD545TTL ==
  note: cf. Modeling_Requirements_36-00-002_v1.2.doc
      | Requirements linked to this component:
      | RQT_LOGOUT_GEN001_IZCD545TTL
      | RQT_LOGOUT_GEN002_IZCD545TTL
      | RQT_LOGOUT_GEN003_IZCD545TTL
      | RQT_LOGOUT_GEN004_IZCD545TTL
      | RQT_LOGOUT_GEN005_IZCD545TTL
      | RQT_LOGOUT_GEN006_IZCD545TTL
      | RQT_LOGOUT_GEN007_IZCD545TTL
  LogicalState : Inport
  SupplyVoltage : Inport  Port=2
  RSA_ServECU_Activation : Renault_Library/Services ECU/Commonly_Used_Blocks/RSA_ServECU_Activation
  RSA_ServECU_EnaOutContact : Renault_Library/Services ECU/Commonly_Used_Blocks/RSA_ServECU_EnaOutContact
  PhysicalOutput_F : Outport
  wires:
  RSA_ServECU_Activation/1 -> RSA_ServECU_EnaOutContact/Enable
  LogicalState/1 -> RSA_ServECU_EnaOutContact/2
  SupplyVoltage/1 -> RSA_ServECU_Activation/1, RSA_ServECU_EnaOutContact/1
  RSA_ServECU_EnaOutContact/1 -> PhysicalOutput_F/1

== system Renault_Library/Services ECU/HW Outputs/RSA_SmartFET ==
  note: cf. Modeling_Requirements_36-00-002_v1.2.doc
      | Requirements linked to this component:
      | RQT_ALOGOUT_GEN001_SmartFET
      | RQT_ALOGOUT_GEN002_SmartFET
      | RQT_ALOGOUT_GEN007_SmartFET
      | RQT_ALOGOUT_ADDGEN001_SmartFET
  PwmRatio : Inport
  PinSupply : Inport  Port=2
  NoSmartProtection : Inport  Port=3
  SupplyVoltage : Inport  Port=4
  RSA_ServECU_Activation : Renault_Library/Services ECU/Commonly_Used_Blocks/RSA_ServECU_Activation
  RSA_ServECU_SmartFET : Renault_Library/Services ECU/Commonly_Used_Blocks/RSA_ServECU_SmartFET
  PhysicalOutput : Outport
  OutputCmd : Outport  Port=2
  wires:
  RSA_ServECU_SmartFET/1 -> PhysicalOutput/1
  SupplyVoltage/1 -> RSA_ServECU_Activation/1
  RSA_ServECU_Activation/1 -> RSA_ServECU_SmartFET/Enable
  PwmRatio/1 -> RSA_ServECU_SmartFET/1
  PinSupply/1 -> RSA_ServECU_SmartFET/2
  NoSmartProtection/1 -> RSA_ServECU_SmartFET/3
  RSA_ServECU_SmartFET/2 -> OutputCmd/1

== system Renault_Library/Services ECU/Mux/RSA_CANFaultManagement ==
  Fault_Management_Allowed : Inport
  Failure : Inport  Port=2
  Signal : Inport  Port=3
  Frame_Status : Inport  Port=4
  ClearDiagnosticInformation : Inport  Port=5
  CANSignal_FaultConfTime : Constant  Value=CANSignal_FaultConfTime
  CANSignal_FaultRehabTime : Constant  Value=CANSignal_FaultRehabTime
  CANSignal_SwitchValue : Constant  Value=CANSignal_SwitchValue
  CANSignal_UnavailableValue : Constant  Value=CANSignal_UnavailableValue
  DataTypeConversion{,1..3} (4 alike) : DataTypeConversion  RndMeth=Zero
      settings: AttributesFormatString=Saturation:%<SaturateOnIntegerOverflow>
  Frame_AbsentValue : Constant  Value=Frame_AbsentValue
  Frame_ConfirmedAbsentValue : Constant  Value=Frame_ConfirmedAbsentValue
  LogicalOperator : Logic  Operator=NOT  OutDataTypeStr=boolean
  LogicalOperator1 : Logic  Operator=OR  OutDataTypeStr=boolean
  LogicalOperator2 : Logic  OutDataTypeStr=boolean
  LogicalOperator3 : Logic  Operator=OR  OutDataTypeStr=boolean
  RSA_CANSignalFailMonitoring : Subsystem  AncestorBlock=AssystemProposal/RSA_CANSignalFailMonitoring  mask(RSA_CANSignalFailMonitoring){ResetValue=0, Ts=SampleTime_C}
      mask prompts: ResetValue "Reset Value"; Ts "SampleTime (s)"
      mask help:
      | Subsystem to confirm failure on signal
      |
      | Inputs:
      | Fault_Management_Allowed (boolean)
      | ClearDiagnosticInformation = reset diag
      | Failure= boolean true when a failure occures
      | Rehab= boolean true when the failure disappears
      | FaultConfirmation_Time = duration of failure to confirm the failure
      | FaultRehab_Time = duration of disappearance to confirm the rehabilitation
      |
      | Output:
      | Failure_flag (boolean) = true if failure confirmed/ false if rehab
      |
      | Version : 01.00
      mask Help:
      | Inputs:
      | Fault_Management_Allowed (boolean)
      | ClearDiagnosticInformation = reset diag
      | Failure= boolean true when a failure occures
      | Rehab= boolean true when the failure disappears
      | FaultConfirmation_Time = duration of failure to confirm the failure
      | FaultRehab_Time = duration of disappearance to confirm the rehabilitation
      | 
      | Output:
      | Failure_flag (boolean) = true if failure confirmed/ false if rehab
      | 
      | Version : 01.00
  RelationalOperator1 : RelationalOperator  Operator===  OutDataTypeStr=boolean  InputSameDT=on
  RelationalOperator4 : RelationalOperator  Operator===  OutDataTypeStr=boolean  InputSameDT=on
  Switch1 : Switch  Criteria=u2 ~= 0  Threshold=0.5  OutDataTypeStr=Inherit: Inherit via back propagation  RndMeth=Zero  SaturateOnIntegerOverflow=on  InputSameDT=on
  Switch_Invalid_Value : Switch  Criteria=u2 ~= 0  OutDataTypeStr=Inherit: Inherit via back propagation  RndMeth=Zero  InputSameDT=on
  UnitDelay1 : UnitDelay  SampleTime=-1
  Signal_out : Outport
  Signal_Unknown : Outport  Port=2
  Signal_Unknown_Confirmed : Outport  Port=3
  wires:
  RelationalOperator1/1 -> LogicalOperator1/1
  RSA_CANSignalFailMonitoring/1(failure_flag) -> LogicalOperator1/2
  RelationalOperator4/1 -> LogicalOperator3/2
  LogicalOperator1/1 -> Signal_Unknown_Confirmed/1, LogicalOperator2/1
  CANSignal_FaultRehabTime/1 -> RSA_CANSignalFailMonitoring/6(FaultRehab_Time)
  Fault_Management_Allowed/1 -> DataTypeConversion2/1
  CANSignal_FaultConfTime/1 -> RSA_CANSignalFailMonitoring/5(FaultConfirmation_Time)
  Frame_AbsentValue/1 -> RelationalOperator4/1
  Frame_ConfirmedAbsentValue/1 -> RelationalOperator1/2
  Frame_Status/1 -> RelationalOperator4/2, RelationalOperator1/1
  CANSignal_UnavailableValue/1 -> Switch_Invalid_Value/3
  Switch1/1 -> Signal_out/1, UnitDelay1/1
  Signal/1 -> DataTypeConversion/1
  Switch_Invalid_Value/1 -> Switch1/1
  CANSignal_SwitchValue/1 -> Switch_Invalid_Value/2
  UnitDelay1/1 -> Switch_Invalid_Value/1
  [Signal] -> Switch1/3
  LogicalOperator2/1 -> Switch1/2
  [Fault_Management_Allowed] -> LogicalOperator2/2
  LogicalOperator3/1 -> Signal_Unknown/1
  ClearDiagnosticInformation/1 -> DataTypeConversion3/1
  LogicalOperator/1 -> RSA_CANSignalFailMonitoring/4(Rehab)
  Failure/1 -> DataTypeConversion1/1
  DataTypeConversion/1 -> [Signal]
  DataTypeConversion1/1 -> LogicalOperator3/1, LogicalOperator/1, RSA_CANSignalFailMonitoring/3(Failure)
  DataTypeConversion2/1 -> [Fault_Management_Allowed], RSA_CANSignalFailMonitoring/1(Fault_Management_Allowed)
  DataTypeConversion3/1 -> RSA_CANSignalFailMonitoring/2(ClearDiagnosticInformation)

== system Renault_Library/Services ECU/Mux/RSA_CANSignalFailMonitoring ==
  Fault_Management_Allowed : Inport
  ClearDiagnosticInformation : Inport  Port=2
  Failure : Inport  Port=3
  Rehab : Inport  Port=4
  FaultConfirmation_Time : Inport  Port=5
  FaultRehab_Time : Inport  Port=6
  FixPtSum2 : Sum  OutDataTypeStr=Inherit: Inherit via back propagation  RndMeth=Zero  InputSameDT=on  LockScale=on
  FixPtSum3 : Sum  OutDataTypeStr=Inherit: Inherit via back propagation  RndMeth=Zero  InputSameDT=on  LockScale=on
  FixPtSwitch2 : Switch  Criteria=u2 ~= 0  Threshold=0.5  OutDataTypeStr=Inherit: Inherit via back propagation  RndMeth=Zero  ZeroCross=off  InputSameDT=on
  FixPtSwitch3 : Switch  Criteria=u2 ~= 0  Threshold=0.5  OutDataTypeStr=Inherit: Inherit via back propagation  RndMeth=Zero  ZeroCross=off  InputSameDT=on
  LogicalOperator1 : Logic  Operator=OR  OutDataTypeStr=boolean
  LogicalOperator2 : Logic  OutDataTypeStr=boolean
  LogicalOperator5 : Logic  Inputs=3  OutDataTypeStr=boolean
  LogicalOperator6 : Logic  Operator=NOT  Inputs=3  OutDataTypeStr=boolean
  Output1 : UnitDelay  SampleTime=-1
  Output2 : UnitDelay  SampleTime=-1
  Output4 : UnitDelay  SampleTime=-1
  RelationalOperator1 : RelationalOperator  Operator=>  OutDataTypeStr=boolean  ZeroCross=off  InputSameDT=on
  RelationalOperator3 : RelationalOperator  Operator=>  OutDataTypeStr=boolean  ZeroCross=off  InputSameDT=on
  ResetValue : Constant  Value=ResetValue  OutDataTypeStr=Inherit: Inherit via back propagation
  Switch4 : Switch  Criteria=u2 ~= 0  Threshold=0.5  RndMeth=Zero  InputSameDT=on
  Switch6 : Switch  Criteria=u2 ~= 0  Threshold=0.5  RndMeth=Zero  InputSameDT=on
  Ts : Constant  Value=Ts  OutDataTypeStr=Inherit: Inherit via back propagation
  false : Constant  Value=false  OutDataTypeStr=boolean
  true : Constant  Value=true  OutDataTypeStr=boolean
  failure_flag : Outport
  wires:
  Output1/1 -> FixPtSum2/1
  Output2/1 -> FixPtSum3/1
  [Ts] -> FixPtSum3/2
  [Ts] -> FixPtSum2/2
  Ts/1 -> [Ts]
  ResetValue/1 -> [Reset]
  FaultRehab_Time/1 -> RelationalOperator3/2
  FaultConfirmation_Time/1 -> RelationalOperator1/2
  [ClearDiagnosticInformation] -> LogicalOperator1/2
  LogicalOperator1/1 -> Switch6/2
  true/1 -> Switch4/1
  Output4/1 -> Switch6/3
  Switch6/1 -> Switch4/3
  false/1 -> Switch6/1
  Switch4/1 -> Output4/1, failure_flag/1
  FixPtSwitch3/1 -> Output2/1, RelationalOperator3/1
  FixPtSum3/1 -> FixPtSwitch3/1
  [Reset] -> FixPtSwitch3/3
  LogicalOperator2/1 -> FixPtSwitch3/2
  Rehab/1 -> LogicalOperator2/1
  [Reset] -> FixPtSwitch2/3
  LogicalOperator6/1 -> LogicalOperator5/1
  LogicalOperator5/1 -> FixPtSwitch2/2
  Failure/1 -> LogicalOperator5/3
  FixPtSwitch2/1 -> Output1/1, RelationalOperator1/1
  FixPtSum2/1 -> FixPtSwitch2/1
  RelationalOperator1/1 -> Switch4/2
  ClearDiagnosticInformation/1 -> [ClearDiagnosticInformation], LogicalOperator6/1
  Fault_Management_Allowed/1 -> LogicalOperator5/2, LogicalOperator2/2
  RelationalOperator3/1 -> LogicalOperator1/1

== system Renault_Library/Services ECU/Mux/RSA_ConvertFromMux ==
  SIGNAL : Inport
      settings: AttributesFormatString=Type : %<OutDataTypeStr>
  Enable : EnablePort  StatesWhenEnabling=reset
  Compare : RelationalOperator  Operator===  OutDataTypeStr=boolean  InputSameDT=on
  Constant : Constant  DisableCoverage=on  Value=0  OutDataTypeStr=Inherit: Inherit via back propagation  SampleTime=-1  PreserveConstantTs=on
  Constant1 : Constant  Value=Offset  OutDataTypeStr=Inherit: Inherit via back propagation  SampleTime=-1
  Constant2 : Constant  Value=Divisor  OutDataTypeStr=Inherit: Inherit via back propagation  SampleTime=-1
  Constant3 : Constant  Value=Factor  OutDataTypeStr=Inherit: Inherit via back propagation  SampleTime=-1
  Constant4 : Constant  OutDataTypeStr=Inherit: Inherit via back propagation  SampleTime=-1
  Constant5 : Constant  Value=Unavailable  OutDataTypeStr=Inherit: Inherit via back propagation  SampleTime=-1
  Divide : Product  Inputs=*/  OutDataTypeStr=Inherit: Inherit via back propagation  RndMeth=Zero  InputSameDT=on
      settings: AttributesFormatString=Saturation:%<SaturateOnIntegerOverflow>
  Product : Product  OutDataTypeStr=Inherit: Inherit via back propagation  RndMeth=Zero  InputSameDT=on
      settings: AttributesFormatString=Saturation:%<SaturateOnIntegerOverflow>
  RelationalOperator2 : RelationalOperator  Operator===  OutDataTypeStr=boolean  InputSameDT=on
  Sum : Sum  OutDataTypeStr=Inherit: Inherit via back propagation  RndMeth=Zero  InputSameDT=on
      settings: AttributesFormatString=Saturation:%<SaturateOnIntegerOverflow>
  Switch : Switch  Criteria=u2 ~= 0  RndMeth=Zero  InputSameDT=on
      settings: AttributesFormatString=Threshold : %<Threshold>\nSaturation:%<SaturateOnIntegerOverflow>
  DATA : Outport  OutputWhenDisabled=reset  InitialOutput=0
      settings: AttributesFormatString=Type : %<OutDataTypeStr>\nInit :%<InitialOutput>
  UNAVAILABLE_SIGNAL : Outport  Port=2  OutputWhenDisabled=reset  InitialOutput=false
      settings: AttributesFormatString=Type : %<OutDataTypeStr>\nInit :%<InitialOutput>
  wires:
  RelationalOperator2/1 -> UNAVAILABLE_SIGNAL/1
  Constant5/1 -> RelationalOperator2/2
  Constant/1 -> Compare/2
  Switch/1 -> Divide/2
  Constant4/1 -> Switch/1
  Compare/1 -> Switch/2
  Divide/1 -> DATA/1
  Constant2/1 -> Compare/1, Switch/3
  Sum/1 -> Divide/1
  Constant1/1 -> Sum/2
  Product/1 -> Sum/1
  Constant3/1 -> Product/2
  SIGNAL/1 -> RelationalOperator2/1, Product/1
